simulink model "chan_550_debug"  (Simulink 7.3)
settings: Solver=ode45  StartTime=0.0  StopTime=10000  RelTol=1e-3
undefined symbols (not defined in the file): pfb_coeff_gen_calc
libraries: xbsIndex_r4 x3811, casper_library_misc x151, xps_library x51, casper_library_delays x46, casper_library_flow_control x33, casper_library_reorder x4, casper_library_ffts_twiddle x2
(part 2 of 41)

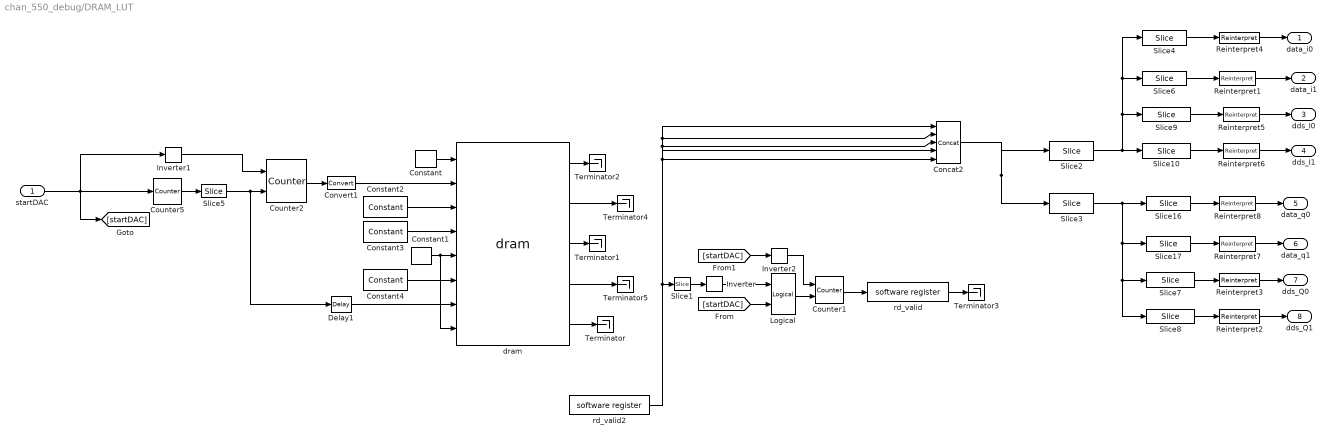
== system chan_550_debug/DRAM_LUT ==
  startDAC : Inport  OutDataType=sfix(16)  OutScaling=2^0
  Concat2 : xbsIndex_r4/Concat  num_inputs=5  dbl_ovrd=off  block_type=concat
      settings: block_version=10.1.3
  Constant : xbsIndex_r4/Constant  arith_type=Boolean  const=0  n_bits=16  bin_pt=14  explicit_period=on  period=1  equ=P=C  opselect=C  inp2=PCIN>>17  opr=+  inp1=P  carry=CIN  dbl_ovrd=off  block_type=constant
  Constant1 : xbsIndex_r4/Constant  arith_type=Boolean  const=1  n_bits=16  bin_pt=14  explicit_period=on  period=1  equ=P=C  opselect=C  inp2=PCIN>>17  opr=+  inp1=P  carry=CIN  dbl_ovrd=off  block_type=constant
  Constant2 : xbsIndex_r4/Constant  arith_type=Unsigned  const=0  n_bits=144  bin_pt=0  explicit_period=on  period=1  equ=P=C  opselect=C  inp2=PCIN>>17  opr=+  inp1=P  carry=CIN  dbl_ovrd=off  block_type=constant
  Constant3 : xbsIndex_r4/Constant  arith_type=Unsigned  const=0  n_bits=36  bin_pt=0  explicit_period=on  period=1  equ=P=C  opselect=C  inp2=PCIN>>17  opr=+  inp1=P  carry=CIN  dbl_ovrd=off  block_type=constant
  Constant4 : xbsIndex_r4/Constant  arith_type=Unsigned  const=0  n_bits=32  bin_pt=0  explicit_period=on  period=1  equ=P=C  opselect=C  inp2=PCIN>>17  opr=+  inp1=P  carry=CIN  dbl_ovrd=off  block_type=constant
  Convert1 : xbsIndex_r4/Convert  arith_type=Unsigned  n_bits=32  bin_pt=0  quantization=Truncate  overflow=Wrap  latency=0  dbl_ovrd=off  pipeline=off  block_type=convert
      settings: block_version=11.4
  Counter1 : xbsIndex_r4/Counter  cnt_type=Free Running  cnt_to=511  operation=Up  start_count=0  cnt_by_val=1  arith_type=Unsigned  n_bits=14  bin_pt=0  load_pin=off  rst=on  en=on  explicit_period=on  period=1  dbl_ovrd=off  use_behavioral_HDL=off  implementation=Fabric  use_rpm=off  block_type=counter
  Counter2 : xbsIndex_r4/Counter  cnt_type=Free Running  cnt_to=512  operation=Up  start_count=0  cnt_by_val=1  arith_type=Unsigned  n_bits=14  bin_pt=0  load_pin=off  rst=on  en=on  explicit_period=on  period=1  dbl_ovrd=off  use_behavioral_HDL=off  implementation=Fabric  use_rpm=off  block_type=counter
  Counter5 : xbsIndex_r4/Counter  cnt_type=Free Running  cnt_to=511  operation=Up  start_count=0  cnt_by_val=1  arith_type=Unsigned  n_bits=1  bin_pt=0  load_pin=off  rst=off  en=on  explicit_period=on  period=1  dbl_ovrd=off  use_behavioral_HDL=off  implementation=Fabric  use_rpm=off  block_type=counter
  Delay1 : xbsIndex_r4/Delay  en=off  latency=1  dbl_ovrd=off  reg_retiming=off  block_type=delay
  Inverter : xbsIndex_r4/Inverter  en=off  latency=0  dbl_ovrd=off  block_type=inv
      settings: block_version=11.4
  Inverter1 : xbsIndex_r4/Inverter  en=off  latency=0  dbl_ovrd=off  block_type=inv
      settings: block_version=11.4
  Inverter2 : xbsIndex_r4/Inverter  en=off  latency=0  dbl_ovrd=off  block_type=inv
      settings: block_version=11.4
  Logical : xbsIndex_r4/Logical  logical_function=AND  inputs=2  en=off  latency=0  precision=Full  arith_type=Unsigned  n_bits=16  bin_pt=0  align_bp=on  dbl_ovrd=off  block_type=logical
      settings: block_version=11.4
  Reinterpret{1..8} (8 alike) : xbsIndex_r4/Reinterpret  force_arith_type=on  arith_type="Signed  (2's comp)"  force_bin_pt=on  bin_pt=15  block_type=reinterpret
      settings: infoedit="Changes signal type without altering the binary representation.   You can changed the signal between signed and unsigned, and relocate the binary point.<P><P>Hardware notes: In hardware this block costs nothing.<P><P>Example:  Suppose the input is 6 bits wide, signed, with 2 fractional bits, and the output is forced to unsigned with 0 fractional bits.  Then an input of -2.0 (1110.00 in binary 2's complement) becomes an output of 56 (111000 in binary)."  block_version=10.1.3
  Slice1 : xbsIndex_r4/Slice  nbits=1  boolean_output=on  mode=Lower Bit Location + Width  bit1=0  base1=MSB of Input  bit0=0  base0=LSB of Input  dbl_ovrd=off  block_type=slice
      settings: block_version=10.1.3
  Slice10 : xbsIndex_r4/Slice  nbits=16  boolean_output=off  mode=Lower Bit Location + Width  bit1=0  base1=MSB of Input  bit0=48  base0=LSB of Input  dbl_ovrd=off  block_type=slice
      settings: block_version=10.1.3
  Slice16 : xbsIndex_r4/Slice  nbits=16  boolean_output=off  mode=Lower Bit Location + Width  bit1=0  base1=MSB of Input  bit0=0  base0=LSB of Input  dbl_ovrd=off  block_type=slice
      settings: block_version=10.1.3
  Slice17 : xbsIndex_r4/Slice  nbits=16  boolean_output=off  mode=Lower Bit Location + Width  bit1=0  base1=LSB of Input  bit0=16  base0=LSB of Input  dbl_ovrd=off  block_type=slice
      settings: block_version=10.1.3
  Slice2 : xbsIndex_r4/Slice  nbits=64  boolean_output=off  mode=Lower Bit Location + Width  bit1=0  base1=MSB of Input  bit0=0  base0=LSB of Input  dbl_ovrd=off  block_type=slice
      settings: block_version=10.1.3
  Slice3 : xbsIndex_r4/Slice  nbits=64  boolean_output=off  mode=Lower Bit Location + Width  bit1=0  base1=MSB of Input  bit0=72  base0=LSB of Input  dbl_ovrd=off  block_type=slice
      settings: block_version=10.1.3
  Slice4 : xbsIndex_r4/Slice  nbits=16  boolean_output=off  mode=Lower Bit Location + Width  bit1=0  base1=MSB of Input  bit0=0  base0=LSB of Input  dbl_ovrd=off  block_type=slice
      settings: block_version=10.1.3
  Slice5 : xbsIndex_r4/Slice  nbits=1  boolean_output=on  mode=Lower Bit Location + Width  bit1=0  base1=MSB of Input  bit0=0  base0=LSB of Input  dbl_ovrd=off  block_type=slice
      settings: block_version=10.1.3
  Slice6 : xbsIndex_r4/Slice  nbits=16  boolean_output=off  mode=Lower Bit Location + Width  bit1=0  base1=LSB of Input  bit0=16  base0=LSB of Input  dbl_ovrd=off  block_type=slice
      settings: block_version=10.1.3
  Slice7 : xbsIndex_r4/Slice  nbits=16  boolean_output=off  mode=Lower Bit Location + Width  bit1=0  base1=MSB of Input  bit0=32  base0=LSB of Input  dbl_ovrd=off  block_type=slice
      settings: block_version=10.1.3
  Slice8 : xbsIndex_r4/Slice  nbits=16  boolean_output=off  mode=Lower Bit Location + Width  bit1=0  base1=MSB of Input  bit0=48  base0=LSB of Input  dbl_ovrd=off  block_type=slice
      settings: block_version=10.1.3
  Slice9 : xbsIndex_r4/Slice  nbits=16  boolean_output=off  mode=Lower Bit Location + Width  bit1=0  base1=MSB of Input  bit0=32  base0=LSB of Input  dbl_ovrd=off  block_type=slice
      settings: block_version=10.1.3
  Terminator{,1..5} (6 alike) : Terminator
  dram : xps_library/dram  Tag=xps:dram  SystemSampleTime=-1  dimm=1  arith_type=Unsigned  bin_pt=0  ip_clock=300  sample_period=1  bram_fifos=off  disable_tag=off  use_sim=off  ram_depth=18  bank_mgt=off  wide_data=off  half_burst=off  use_sniffer=on
      link override convert_data_in: arith_type=Unsigned  n_bits=144  bin_pt=0
      link override convert_wr_be: n_bits=18
      link override ddc256_4x_nofir_snap_DRAM_LUT_dram_Mem_Rd_Dout: n_bits=144
      link override ddc256_4x_snap2_DRAM_LUT_dram_Mem_Rd_Dout: n_bits=144
      link override ddc256_4x_snap_fir_DRAM_LUT_dram_Mem_Rd_Dout: n_bits=144
      link override force_rd_dout: arith_type=Unsigned  bin_pt=0
      link override sim_wrapper/dram_sim: sim_method=Inactive  engine_block=ModelSim
  rd_valid : xps_library/software register  Tag=xps:sw_reg  SystemSampleTime=-1  io_dir=To Processor  arith_type=Unsigned  bitwidth=32  bin_pt=0  sample_period=1  gw_name=chan_550_debug_DRAM_LUT_rd_valid_user_data_in
      link override DAC_Final_monitor2_user_data_out: arith_type="Signed  (2's comp)"  n_bits=32  bin_pt=0  period=1
      link override DAC_Final_sync_i_user_data_out: arith_type="Signed  (2's comp)"  n_bits=32  bin_pt=0  period=1
      link override Single_Sine_wave_sync_i_user_data_out: arith_type="Signed  (2's comp)"  n_bits=32  bin_pt=0  period=1
      link override adcToDac2_startDAC_user_data_out: arith_type=Unsigned  n_bits=32  bin_pt=0  period=1
      link override adcToDac4_startDAC_user_data_out: arith_type=Unsigned  n_bits=32  bin_pt=0  period=1
      link override adcToDac5_startDAC_user_data_out: arith_type=Unsigned  n_bits=32  bin_pt=0  period=1
      link override adcToDac6_startDAC_user_data_out: arith_type=Unsigned  n_bits=32  bin_pt=0  period=1
      link override adcToDac7_startDAC_user_data_out: arith_type=Unsigned  n_bits=32  bin_pt=0  period=1
      link override adcToDac_startDAC_user_data_out: arith_type=Unsigned  n_bits=32  bin_pt=0  period=1
      link override adcToDac_sync_i_user_data_out: arith_type="Signed  (2's comp)"  n_bits=32  bin_pt=0  period=1
      link override config10_demo_startDAC_user_data_out: arith_type=Unsigned  n_bits=32  bin_pt=0  period=1
      link override convert: arith_type="Signed  (2's comp)"  n_bits=32  bin_pt=0
      link override dac5681_10_1_software_register_user_data_out: arith_type="Signed  (2's comp)"  n_bits=32  bin_pt=0  period=1
      link override dacADCdemo1_startDAC_user_data_out: arith_type=Unsigned  n_bits=32  bin_pt=0  period=1
      link override dacAdcSnap_startDAC_user_data_out: arith_type=Unsigned  n_bits=32  bin_pt=0  period=1
      link override dacToAdcSnapshot_startDAC_user_data_out: arith_type=Unsigned  n_bits=32  bin_pt=0  period=1
      link override dac_bram_new_block_test_v5_monitor2_user_data_out: arith_type="Signed  (2's comp)"  n_bits=32  bin_pt=0  period=1
      link override dac_bram_new_block_test_v6_monitor2_user_data_out: arith_type="Signed  (2's comp)"  n_bits=32  bin_pt=0  period=1
      link override dac_bram_new_block_test_v7_monitor2_user_data_out: arith_type="Signed  (2's comp)"  n_bits=32  bin_pt=0  period=1
      link override dac_bram_new_block_test_v8_monitor2_user_data_out: arith_type="Signed  (2's comp)"  n_bits=32  bin_pt=0  period=1
      link override dac_bram_new_block_test_v9_monitor2_user_data_out: arith_type="Signed  (2's comp)"  n_bits=32  bin_pt=0  period=1
      link override dac_final_200mhz_sync_i_user_data_out: arith_type="Signed  (2's comp)"  n_bits=32  bin_pt=0  period=1
      link override dac_final_2_sync_i_user_data_out: arith_type="Signed  (2's comp)"  n_bits=32  bin_pt=0  period=1
      link override dac_final_550MHz_V2_sync_i_user_data_out: arith_type="Signed  (2's comp)"  n_bits=32  bin_pt=0  period=1
      link override dac_final_550MHz_sync_i_user_data_out: arith_type="Signed  (2's comp)"  n_bits=32  bin_pt=0  period=1
      link override dac_final_550mhz_delay_sync_i_user_data_out: arith_type="Signed  (2's comp)"  n_bits=32  bin_pt=0  period=1
      link override dac_final_550mhz_new2_sync_i_user_data_out: arith_type="Signed  (2's comp)"  n_bits=32  bin_pt=0  period=1
      link override dac_final_550mhz_new_sync_i_user_data_out: arith_type="Signed  (2's comp)"  n_bits=32  bin_pt=0  period=1
      link override dac_final_550mhz_sync_i_user_data_out: arith_type="Signed  (2's comp)"  n_bits=32  bin_pt=0  period=1
      link override dac_final_sync_i_user_data_out: arith_type="Signed  (2's comp)"  n_bits=32  bin_pt=0  period=1
      link override dac_new_copy2_sync_i_user_data_out: arith_type="Signed  (2's comp)"  n_bits=32  bin_pt=0  period=1
      link override dac_new_copy3_sync_i_user_data_out: arith_type="Signed  (2's comp)"  n_bits=32  bin_pt=0  period=1
      link override dac_new_copy4_sync_i_user_data_out: arith_type="Signed  (2's comp)"  n_bits=32  bin_pt=0  period=1
      link override dac_new_copy_sync_i_user_data_out: arith_type="Signed  (2's comp)"  n_bits=32  bin_pt=0  period=1
      link override dac_new_sync_i_user_data_out: arith_type="Signed  (2's comp)"  n_bits=32  bin_pt=0  period=1
      link override ddc256_4x_b_startDAC_user_data_out: arith_type=Unsigned  n_bits=32  bin_pt=0  period=1
      link override ddc256_4x_c_startDAC_user_data_out: arith_type=Unsigned  n_bits=32  bin_pt=0  period=1
      link override ddc256_4x_nofir_snap_startDAC_user_data_out: arith_type=Unsigned  n_bits=32  bin_pt=0  period=1
      link override ddc256_4x_nofir_startDAC_user_data_out: arith_type=Unsigned  n_bits=32  bin_pt=0  period=1
      link override ddc256_4x_snap2_startDAC_user_data_out: arith_type=Unsigned  n_bits=32  bin_pt=0  period=1
      link override ddc256_4x_snap_fir_startDAC_user_data_out: arith_type=Unsigned  n_bits=32  bin_pt=0  period=1
      link override ddc256_low_sim_startDAC_user_data_out: arith_type=Unsigned  n_bits=32  bin_pt=0  period=1
      link override ddc256_low_startDAC_user_data_out: arith_type=Unsigned  n_bits=32  bin_pt=0  period=1
      link override ddc256_nofir_sim_startDAC_user_data_out: arith_type=Unsigned  n_bits=32  bin_pt=0  period=1
      link override ddc2_startDAC_user_data_out: arith_type=Unsigned  n_bits=32  bin_pt=0  period=1
      link override ddcFull_startDAC_user_data_out: arith_type=Unsigned  n_bits=32  bin_pt=0  period=1
      link override ddcQDR_noise_startDAC_user_data_out: arith_type=Unsigned  n_bits=32  bin_pt=0  period=1
      link override ddc_startDAC_user_data_out: arith_type=Unsigned  n_bits=32  bin_pt=0  period=1
      link override fff_11_complex_startDAC_user_data_out: arith_type=Unsigned  n_bits=32  bin_pt=0  period=1
      link override fff_11_withoutfir_startDAC_user_data_out: arith_type=Unsigned  n_bits=32  bin_pt=0  period=1
      link override fff_32bit_11_withoutfir_new_fft_startDAC_user_data_out: arith_type=Unsigned  n_bits=32  bin_pt=0  period=1
      link override fff_32bit_11_withoutfir_startDAC_user_data_out: arith_type=Unsigned  n_bits=32  bin_pt=0  period=1
      link override fff_test_1_startDAC_user_data_out: arith_type=Unsigned  n_bits=32  bin_pt=0  period=1
      link override fff_working_11_fft_vacc_const4_startDAC_user_data_out: arith_type=Unsigned  n_bits=32  bin_pt=0  period=1
      link override fff_working_11_fft_vacc_long_2_startDAC_user_data_out: arith_type=Unsigned  n_bits=32  bin_pt=0  period=1
      link override fff_working_11_fft_vacc_long_startDAC_user_data_out: arith_type=Unsigned  n_bits=32  bin_pt=0  period=1
      link override fff_working_11_fft_vacc_startDAC_user_data_out: arith_type=Unsigned  n_bits=32  bin_pt=0  period=1
      link override fff_working_11_final_startDAC_user_data_out: arith_type=Unsigned  n_bits=32  bin_pt=0  period=1
      link override ffff_11_startDAC_user_data_out: arith_type=Unsigned  n_bits=32  bin_pt=0  period=1
      link override fftStandard_startDAC_user_data_out: arith_type=Unsigned  n_bits=32  bin_pt=0  period=1
      link override mkid_dac_test_input_data_i_user_data_out: arith_type="Signed  (2's comp)"  n_bits=32  bin_pt=0  period=1
      link override mkid_dac_test_with_ROM_input_data_i_user_data_out: arith_type="Signed  (2's comp)"  n_bits=32  bin_pt=0  period=1
      link override mkid_dac_test_with_ROM_v2_input_data_i_user_data_out: arith_type="Signed  (2's comp)"  n_bits=32  bin_pt=0  period=1
      link override mkid_dac_test_with_ROM_v3_input_data_i_user_data_out: arith_type="Signed  (2's comp)"  n_bits=32  bin_pt=0  period=1
      link override mkid_dac_test_with_ROM_v5_input_data_i_user_data_out: arith_type="Signed  (2's comp)"  n_bits=32  bin_pt=0  period=1
      link override mkid_dac_test_with_ROM_v6_input_data_i_user_data_out: arith_type="Signed  (2's comp)"  n_bits=32  bin_pt=0  period=1
      link override mkid_fft_noloss_11_startDAC_user_data_out: arith_type=Unsigned  n_bits=32  bin_pt=0  period=1
      link override mkid_fft_simulation_startDAC_user_data_out: arith_type=Unsigned  n_bits=32  bin_pt=0  period=1
      link override single_Sine_wave_copy2_100mhz_sync_i_user_data_out: arith_type="Signed  (2's comp)"  n_bits=32  bin_pt=0  period=1
      link override single_Sine_wave_copy2_sync_i_user_data_out: arith_type="Signed  (2's comp)"  n_bits=32  bin_pt=0  period=1
      link override single_Sine_wave_sync_i_user_data_out: arith_type="Signed  (2's comp)"  n_bits=32  bin_pt=0  period=1
  rd_valid2 : xps_library/software register  Tag=xps:sw_reg  SystemSampleTime=-1  io_dir=To Processor  arith_type=Unsigned  bitwidth=32  bin_pt=0  sample_period=1  gw_name=chan_550_debug_DRAM_LUT_rd_valid_user_data_in
      link override DAC_Final_monitor2_user_data_out: arith_type="Signed  (2's comp)"  n_bits=32  bin_pt=0  period=1
      link override DAC_Final_sync_i_user_data_out: arith_type="Signed  (2's comp)"  n_bits=32  bin_pt=0  period=1
      link override Single_Sine_wave_sync_i_user_data_out: arith_type="Signed  (2's comp)"  n_bits=32  bin_pt=0  period=1
      link override adcToDac2_startDAC_user_data_out: arith_type=Unsigned  n_bits=32  bin_pt=0  period=1
      link override adcToDac4_startDAC_user_data_out: arith_type=Unsigned  n_bits=32  bin_pt=0  period=1
      link override adcToDac5_startDAC_user_data_out: arith_type=Unsigned  n_bits=32  bin_pt=0  period=1
      link override adcToDac6_startDAC_user_data_out: arith_type=Unsigned  n_bits=32  bin_pt=0  period=1
      link override adcToDac7_startDAC_user_data_out: arith_type=Unsigned  n_bits=32  bin_pt=0  period=1
      link override adcToDac_startDAC_user_data_out: arith_type=Unsigned  n_bits=32  bin_pt=0  period=1
      link override adcToDac_sync_i_user_data_out: arith_type="Signed  (2's comp)"  n_bits=32  bin_pt=0  period=1
      link override config10_demo_startDAC_user_data_out: arith_type=Unsigned  n_bits=32  bin_pt=0  period=1
      link override convert: arith_type="Signed  (2's comp)"  n_bits=32  bin_pt=0
      link override dac5681_10_1_software_register_user_data_out: arith_type="Signed  (2's comp)"  n_bits=32  bin_pt=0  period=1
      link override dacADCdemo1_startDAC_user_data_out: arith_type=Unsigned  n_bits=32  bin_pt=0  period=1
      link override dacAdcSnap_startDAC_user_data_out: arith_type=Unsigned  n_bits=32  bin_pt=0  period=1
      link override dacToAdcSnapshot_startDAC_user_data_out: arith_type=Unsigned  n_bits=32  bin_pt=0  period=1
      link override dac_bram_new_block_test_v5_monitor2_user_data_out: arith_type="Signed  (2's comp)"  n_bits=32  bin_pt=0  period=1
      link override dac_bram_new_block_test_v6_monitor2_user_data_out: arith_type="Signed  (2's comp)"  n_bits=32  bin_pt=0  period=1
      link override dac_bram_new_block_test_v7_monitor2_user_data_out: arith_type="Signed  (2's comp)"  n_bits=32  bin_pt=0  period=1
      link override dac_bram_new_block_test_v8_monitor2_user_data_out: arith_type="Signed  (2's comp)"  n_bits=32  bin_pt=0  period=1
      link override dac_bram_new_block_test_v9_monitor2_user_data_out: arith_type="Signed  (2's comp)"  n_bits=32  bin_pt=0  period=1
      link override dac_final_200mhz_sync_i_user_data_out: arith_type="Signed  (2's comp)"  n_bits=32  bin_pt=0  period=1
      link override dac_final_2_sync_i_user_data_out: arith_type="Signed  (2's comp)"  n_bits=32  bin_pt=0  period=1
      link override dac_final_550MHz_V2_sync_i_user_data_out: arith_type="Signed  (2's comp)"  n_bits=32  bin_pt=0  period=1
      link override dac_final_550MHz_sync_i_user_data_out: arith_type="Signed  (2's comp)"  n_bits=32  bin_pt=0  period=1
      link override dac_final_550mhz_delay_sync_i_user_data_out: arith_type="Signed  (2's comp)"  n_bits=32  bin_pt=0  period=1
      link override dac_final_550mhz_new2_sync_i_user_data_out: arith_type="Signed  (2's comp)"  n_bits=32  bin_pt=0  period=1
      link override dac_final_550mhz_new_sync_i_user_data_out: arith_type="Signed  (2's comp)"  n_bits=32  bin_pt=0  period=1
      link override dac_final_550mhz_sync_i_user_data_out: arith_type="Signed  (2's comp)"  n_bits=32  bin_pt=0  period=1
      link override dac_final_sync_i_user_data_out: arith_type="Signed  (2's comp)"  n_bits=32  bin_pt=0  period=1
      link override dac_new_copy2_sync_i_user_data_out: arith_type="Signed  (2's comp)"  n_bits=32  bin_pt=0  period=1
      link override dac_new_copy3_sync_i_user_data_out: arith_type="Signed  (2's comp)"  n_bits=32  bin_pt=0  period=1
      link override dac_new_copy4_sync_i_user_data_out: arith_type="Signed  (2's comp)"  n_bits=32  bin_pt=0  period=1
      link override dac_new_copy_sync_i_user_data_out: arith_type="Signed  (2's comp)"  n_bits=32  bin_pt=0  period=1
      link override dac_new_sync_i_user_data_out: arith_type="Signed  (2's comp)"  n_bits=32  bin_pt=0  period=1
      link override ddc256_4x_b_startDAC_user_data_out: arith_type=Unsigned  n_bits=32  bin_pt=0  period=1
      link override ddc256_4x_c_startDAC_user_data_out: arith_type=Unsigned  n_bits=32  bin_pt=0  period=1
      link override ddc256_4x_nofir_snap_startDAC_user_data_out: arith_type=Unsigned  n_bits=32  bin_pt=0  period=1
      link override ddc256_4x_nofir_startDAC_user_data_out: arith_type=Unsigned  n_bits=32  bin_pt=0  period=1
      link override ddc256_4x_snap2_startDAC_user_data_out: arith_type=Unsigned  n_bits=32  bin_pt=0  period=1
      link override ddc256_4x_snap_fir_startDAC_user_data_out: arith_type=Unsigned  n_bits=32  bin_pt=0  period=1
      link override ddc256_low_sim_startDAC_user_data_out: arith_type=Unsigned  n_bits=32  bin_pt=0  period=1
      link override ddc256_low_startDAC_user_data_out: arith_type=Unsigned  n_bits=32  bin_pt=0  period=1
      link override ddc256_nofir_sim_startDAC_user_data_out: arith_type=Unsigned  n_bits=32  bin_pt=0  period=1
      link override ddc2_startDAC_user_data_out: arith_type=Unsigned  n_bits=32  bin_pt=0  period=1
      link override ddcFull_startDAC_user_data_out: arith_type=Unsigned  n_bits=32  bin_pt=0  period=1
      link override ddcQDR_noise_startDAC_user_data_out: arith_type=Unsigned  n_bits=32  bin_pt=0  period=1
      link override ddc_startDAC_user_data_out: arith_type=Unsigned  n_bits=32  bin_pt=0  period=1
      link override fff_11_complex_startDAC_user_data_out: arith_type=Unsigned  n_bits=32  bin_pt=0  period=1
      link override fff_11_withoutfir_startDAC_user_data_out: arith_type=Unsigned  n_bits=32  bin_pt=0  period=1
      link override fff_32bit_11_withoutfir_new_fft_startDAC_user_data_out: arith_type=Unsigned  n_bits=32  bin_pt=0  period=1
      link override fff_32bit_11_withoutfir_startDAC_user_data_out: arith_type=Unsigned  n_bits=32  bin_pt=0  period=1
      link override fff_test_1_startDAC_user_data_out: arith_type=Unsigned  n_bits=32  bin_pt=0  period=1
      link override fff_working_11_fft_vacc_const4_startDAC_user_data_out: arith_type=Unsigned  n_bits=32  bin_pt=0  period=1
      link override fff_working_11_fft_vacc_long_2_startDAC_user_data_out: arith_type=Unsigned  n_bits=32  bin_pt=0  period=1
      link override fff_working_11_fft_vacc_long_startDAC_user_data_out: arith_type=Unsigned  n_bits=32  bin_pt=0  period=1
      link override fff_working_11_fft_vacc_startDAC_user_data_out: arith_type=Unsigned  n_bits=32  bin_pt=0  period=1
      link override fff_working_11_final_startDAC_user_data_out: arith_type=Unsigned  n_bits=32  bin_pt=0  period=1
      link override ffff_11_startDAC_user_data_out: arith_type=Unsigned  n_bits=32  bin_pt=0  period=1
      link override fftStandard_startDAC_user_data_out: arith_type=Unsigned  n_bits=32  bin_pt=0  period=1
      link override mkid_dac_test_input_data_i_user_data_out: arith_type="Signed  (2's comp)"  n_bits=32  bin_pt=0  period=1
      link override mkid_dac_test_with_ROM_input_data_i_user_data_out: arith_type="Signed  (2's comp)"  n_bits=32  bin_pt=0  period=1
      link override mkid_dac_test_with_ROM_v2_input_data_i_user_data_out: arith_type="Signed  (2's comp)"  n_bits=32  bin_pt=0  period=1
      link override mkid_dac_test_with_ROM_v3_input_data_i_user_data_out: arith_type="Signed  (2's comp)"  n_bits=32  bin_pt=0  period=1
      link override mkid_dac_test_with_ROM_v5_input_data_i_user_data_out: arith_type="Signed  (2's comp)"  n_bits=32  bin_pt=0  period=1
      link override mkid_dac_test_with_ROM_v6_input_data_i_user_data_out: arith_type="Signed  (2's comp)"  n_bits=32  bin_pt=0  period=1
      link override mkid_fft_noloss_11_startDAC_user_data_out: arith_type=Unsigned  n_bits=32  bin_pt=0  period=1
      link override mkid_fft_simulation_startDAC_user_data_out: arith_type=Unsigned  n_bits=32  bin_pt=0  period=1
      link override single_Sine_wave_copy2_100mhz_sync_i_user_data_out: arith_type="Signed  (2's comp)"  n_bits=32  bin_pt=0  period=1
      link override single_Sine_wave_copy2_sync_i_user_data_out: arith_type="Signed  (2's comp)"  n_bits=32  bin_pt=0  period=1
      link override single_Sine_wave_sync_i_user_data_out: arith_type="Signed  (2's comp)"  n_bits=32  bin_pt=0  period=1
  data_i0 : Outport
  data_i1 : Outport  Port=2
  dds_I0 : Outport  Port=3
  dds_I1 : Outport  Port=4
  data_q0 : Outport  Port=5
  data_q1 : Outport  Port=6
  dds_Q0 : Outport  Port=7
  dds_Q1 : Outport  Port=8
  wires:
  Constant/1 -> dram/1
  Constant1/1 -> dram/8, dram/5
  Constant2/1 -> dram/3
  Constant3/1 -> dram/4
  Slice5/1 -> Counter2/2, Delay1/1
  dram/4 -> Terminator5/1
  Constant4/1 -> dram/6
  Slice4/1 -> Reinterpret4/1
  Slice6/1 -> Reinterpret1/1
  Slice9/1 -> Reinterpret5/1
  Slice10/1 -> Reinterpret6/1
  Reinterpret4/1 -> data_i0/1
  Reinterpret1/1 -> data_i1/1
  Reinterpret5/1 -> dds_I0/1
  Reinterpret6/1 -> dds_I1/1
  Reinterpret8/1 -> data_q0/1
  Reinterpret7/1 -> data_q1/1
  Reinterpret3/1 -> dds_Q0/1
  Reinterpret2/1 -> dds_Q1/1
  startDAC/1 -> Counter5/1, [startDAC], Inverter1/1
  Counter5/1 -> Slice5/1
  Inverter/1 -> Logical/1
  [startDAC] -> Logical/2
  Convert1/1 -> dram/2
  Slice16/1 -> Reinterpret8/1
  Slice17/1 -> Reinterpret7/1
  Slice7/1 -> Reinterpret3/1
  Slice8/1 -> Reinterpret2/1
  Slice2/1 -> Slice10/1, Slice9/1, Slice6/1, Slice4/1
  Slice3/1 -> Slice16/1, Slice17/1, Slice7/1, Slice8/1
  Counter1/1 -> rd_valid/1
  Logical/1 -> Counter1/2
  [startDAC] -> Inverter2/1
  Inverter2/1 -> Counter1/1
  dram/2 -> Terminator4/1
  Counter2/1 -> Convert1/1
  Inverter1/1 -> Counter2/1
  dram/5 -> Terminator/1
  dram/3 -> Terminator1/1
  dram/1 -> Terminator2/1
  rd_valid/1 -> Terminator3/1
  Delay1/1 -> dram/7
  rd_valid2/1 -> Slice1/1, Concat2/1, Concat2/2, Concat2/3, Concat2/4, Concat2/5
  Slice1/1 -> Inverter/1
  Concat2/1 -> Slice2/1, Slice3/1
